simulink model "non_linear_MBS_PF_final"  (R2021b)
settings: Solver=ode15s  StartTime=0  StopTime=SimTime  FixedStep=.00001  RelTol=1e-3
values from neighbouring files (symbols this model uses):
  A_ratio = 1.41    [non_linear_param_MBS_PF_final.m]    (also in non_linear_param_final.m)
  Annulus_area = Bore_area-Rod_area    (= 2356.19449019)    [non_linear_param_MBS_PF_final.m]    (also in non_linear_param_final.m)
  beta_F = 12000e5    (= 1200000000)    [non_linear_param_MBS_PF_final.m]    (also in non_linear_param_final.m)
  Bore_area = pi/4*Bore_diameter^2    (= 3318.30724035)    [non_linear_param_MBS_PF_final.m]    (also in non_linear_param_final.m)
  cyl_c_end = 9e8    (= 900000000)    [non_linear_param_MBS_PF_final.m]    (also in non_linear_param_final.m)
  cyl_c_visc = 1500    [non_linear_param_MBS_PF_final.m]    (also in non_linear_param_final.m)
  cyl_CH_stat = 1/50    (= 0.02)    [non_linear_param_MBS_PF_final.m]    (also in non_linear_param_final.m)
  cyl_F_coul = 75    [non_linear_param_MBS_PF_final.m]    (also in non_linear_param_final.m)
  cyl_FH_stat = 500    [non_linear_param_MBS_PF_final.m]    (also in non_linear_param_final.m)
  cyl_gamma = 250    [non_linear_param_MBS_PF_final.m]    (also in non_linear_param_final.m)
  cyl_k_end = 6e9    (= 6000000000)    [non_linear_param_MBS_PF_final.m]    (also in non_linear_param_final.m)
  cyl_KLi = 0    [non_linear_param_MBS_PF_final.m]    (also in non_linear_param_final.m)
  d_eff_long = sqrt(2*J_sec_long*k_eff_long)    [non_linear_param_MBS_PF_final.m]    (also in non_linear_param_final.m)
  d_eff_short = sqrt(2*J_sec_short*k_eff_short)    [non_linear_param_MBS_PF_final.m]    (also in non_linear_param_final.m)
  DB = 0    [non_linear_param_MBS_PF_final.m]    (also in non_linear_param_final.m = -0.114)
  DR = 1    [non_linear_param_MBS_PF_final.m]    (also in non_linear_param_final.m)
  dr_dcv = 0.7    [non_linear_param_MBS_PF_final.m]    (also in non_linear_param_final.m)
  Eff_Bulk_On = 1    [non_linear_param_MBS_PF_final.m]    (also in non_linear_param_final.m)
  eta_air = 0.7e-2    (= 0.007)    [non_linear_param_MBS_PF_final.m]    (also in non_linear_param_final.m)
  k_cv = 15    [non_linear_param_MBS_PF_final.m]    (also in non_linear_param_final.m)
  k_DCV_PA = 7.85    [non_linear_param_MBS_PF_final.m]    (also in non_linear_param_final.m)
  k_DCV_PB = 7.55    [non_linear_param_MBS_PF_final.m]    (also in non_linear_param_final.m)
  k_DCV_T = 7.85    [non_linear_param_MBS_PF_final.m]    (also in non_linear_param_final.m)
  k_eff_long = E*I/I_sec_long    (= 3050381.94041)    [non_linear_param_MBS_PF_final.m]    (also in non_linear_param_final.m)
  k_eff_short = E*I/I_sec_short    (= 13451432.5427)    [non_linear_param_MBS_PF_final.m]    (also in non_linear_param_final.m)
  k_ocv = 15    [non_linear_param_MBS_PF_final.m]    (also in non_linear_param_final.m)
  K_oil = 1.5e4*0+12000    (= 12000)    [non_linear_param_MBS_PF_final.m]    (also in non_linear_param_final.m)
  k_tanh = 0.005    [non_linear_param_MBS_PF_final.m]    (also in non_linear_param_final.m)
  kappa_air = 1.4    [non_linear_param_MBS_PF_final.m]    (also in non_linear_param_final.m)
  kHP = C*kF_pf_Up    (= 2.90878e-12)    [non_linear_param_MBS_PF_final.m]
  kI = (0.54*ku)/Ti    (= 0.048)    [non_linear_param_MBS_PF_final.m]
  kP = 0.45*ku    (= 0.018)    [non_linear_param_MBS_PF_final.m]
  kq_xS_PB = 4.8946e-04    (= 0.00048946)    [non_linear_param_MBS_PF_final.m]
  KR = 1    [non_linear_param_MBS_PF_final.m]    (also in non_linear_param_final.m)
  mu_ocv = 10    [non_linear_param_MBS_PF_final.m]    (also in non_linear_param_final.m = 3)
  muC = (Bore_diameter^2)/(Bore_diameter^2-Rod_diameter^2)    (= 1.40833333333)    [non_linear_param_MBS_PF_final.m]
  OL_pa = -0.114    [non_linear_param_MBS_PF_final.m]    (also in non_linear_param_final.m)
  OL_pb = 0.114    [non_linear_param_MBS_PF_final.m]    (also in non_linear_param_final.m)
  p0_A = 62.79    [non_linear_param_MBS_PF_final.m]    (also in non_linear_param_final.m)
  p0_A1 = 1.08    [non_linear_param_MBS_PF_final.m]    (also in non_linear_param_final.m)
  p0_B = 1.213    [non_linear_param_MBS_PF_final.m]    (also in non_linear_param_final.m)
  p_cv_fo = 1    [non_linear_param_MBS_PF_final.m]    (also in non_linear_param_final.m)
  p_cv_set = 2.8    [non_linear_param_MBS_PF_final.m]    (also in non_linear_param_final.m)
  p_ocv_fo = 350    [non_linear_param_MBS_PF_final.m]    (also in non_linear_param_final.m)
  p_ocv_set = 196    [non_linear_param_MBS_PF_final.m]    (also in non_linear_param_final.m = 150)
  p_pc_set = 7    [non_linear_param_MBS_PF_final.m]    (also in non_linear_param_final.m)
  p_R = 1.1    [non_linear_param_MBS_PF_final.m]    (also in non_linear_param_final.m)
  p_S = 180    [non_linear_param_MBS_PF_final.m]    (also in non_linear_param_final.m)
  patm = 1.013e5    (= 101300)    [non_linear_param_MBS_PF_final.m]    (also in non_linear_param_final.m)
  Stroke = 500    [non_linear_param_MBS_PF_final.m]    (also in non_linear_param_final.m)
  t0 = 3    [non_linear_param_MBS_PF_final.m]    (also in non_linear_param_final.m = 1)
  tau_ocv = 0.0889*0+0.001    (= 0.001)    [non_linear_param_MBS_PF_final.m]    (also in non_linear_param_final.m)
  tau_pc = tau_ocv    (= 0.001)    [non_linear_param_MBS_PF_final.m]    (also in non_linear_param_final.m)
  tHP = tF_pf_Up/(wn_pf)    (= 0.114613180516)    [non_linear_param_MBS_PF_final.m]
  tStepDown = 4.7*0+7.5    (= 7.5)    [non_linear_param_MBS_PF_final.m]    (also in non_linear_param_final.m = 4.6)
  tStepUp = 10.0    (= 10)    [non_linear_param_MBS_PF_final.m]    (also in non_linear_param_final.m = 6.2)
  tWait = 5.0    (= 5)    [non_linear_param_MBS_PF_final.m]    (also in non_linear_param_final.m = 8)
  UL_at = -0.05    [non_linear_param_MBS_PF_final.m]    (also in non_linear_param_final.m)
  UL_bt = 0.05    [non_linear_param_MBS_PF_final.m]    (also in non_linear_param_final.m)
  Up = 1    [non_linear_param_MBS_PF_final.m]    (also in non_linear_param_final.m)
  uS_sp = -0.4    [non_linear_param_MBS_PF_final.m]    (also in non_linear_param_final.m = -0.55)
  V_CH_A = 1.0e-03    (= 0.001)    [non_linear_param_MBS_PF_final.m]    (also in non_linear_param_final.m)
  V_CH_A1 = 0.1e-03    (= 0.0001)    [non_linear_param_MBS_PF_final.m]    (also in non_linear_param_final.m)
  V_CH_B = 1.25e-03    (= 0.00125)    [non_linear_param_MBS_PF_final.m]    (also in non_linear_param_final.m)
  wn_dcv = 30    [non_linear_param_MBS_PF_final.m]    (also in non_linear_param_final.m)
undefined symbols (not defined in the file or in its folder): sigbuilder_block, smiData, uS0, X, Y, Z
libraries: sm_lib x189, nesl_utility x4, eeGeneralControl x2
(part 1 of 6)
settings Model: Creator=Zygis  LastModifiedBy=danielh
settings SolverCC: ZeroCrossAlgorithm=Adaptive  AlgebraicLoopSolver=TrustRegion
settings DataIOCC: ExternalInput=getinputstruct(report1)  InitialState=getstatestruct(report1)  ReturnWorkspaceOutputs=off  Refine=2
settings DebuggingCC: IgnoredZcDiagnostic=none  UniqueDataStoreMsg=error  SFMachineParentedDataDiag=warning  UnderSpecifiedDimensionMsg=warning  ArithmeticOperatorsInVariantConditions=warning
settings HardwareCC: TargetBitPerPointer=64  TargetBitPerSizeT=64  TargetBitPerPtrDiffT=64  TargetLongLongMode=on  TargetIntDivRoundTo=Zero  TargetEndianess=LittleEndian  TargetWordSize=64  TargetHWDeviceType=MATLAB Host  ProdEqTarget=off
settings SFSimCC: SimUseLocalCustomCode=off  CustomCodeUndefinedFunction=UseInterfaceOnly
settings RTWCC: Description=Rapid Simulation Target  SystemTargetFile=rsim.tlc  TemplateMakefile=rsim_default_tmf  GenerateReport=on  RTWUseLocalCustomCode=off
settings RSimTargetCC: TargetLangStandard=C99 (ISO)
settings DiagnosticsConfigSet: SimMechanicsJointTargetOverSpecification=warning

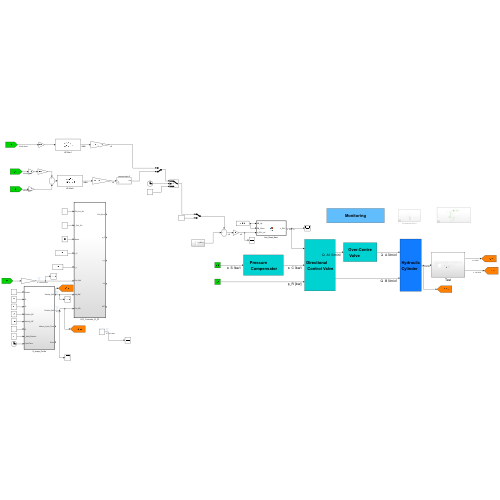
[thumbnail: Simulink's stored preview of the top-level diagram]
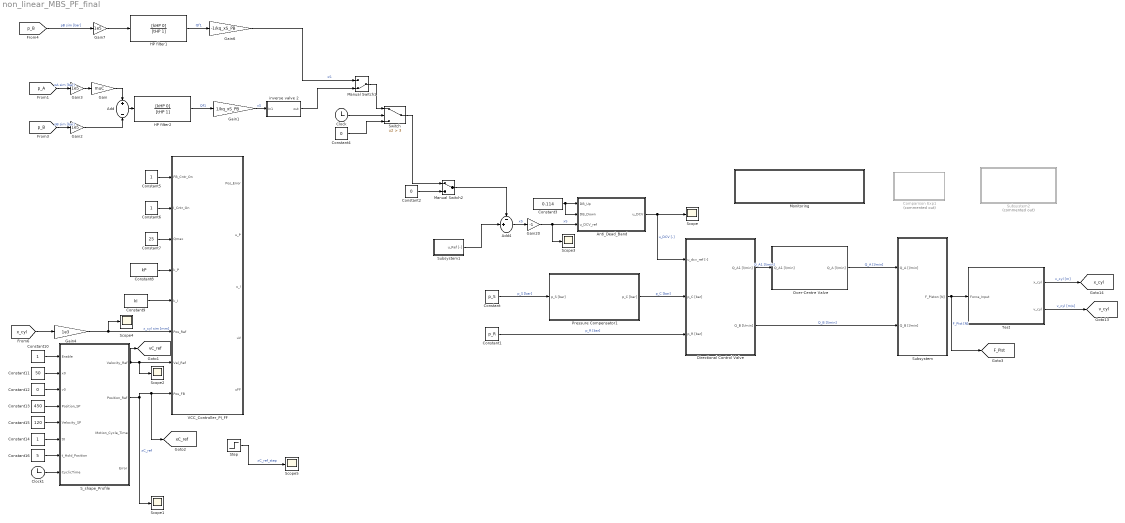
== system non_linear_MBS_PF_final ==
  Add : Sum  Inputs=+-
  Add4 : Sum  Inputs=-+|
  Anti_Dead_Band : MATLAB Function  TreatAsAtomicUnit=on
  Clock : Clock
  Clock1 : Clock
  Comparison Exp1 : Subsystem  Commented=on
  Constant : Constant  Value=p_S
  Constant1 : Constant  Value=p_R
  Constant10 : Constant  OutDataTypeStr=boolean
  Constant11 : Constant  Value=50
  Constant12 : Constant  Value=0
  Constant13 : Constant  Value=450
  Constant14 : Constant
  Constant15 : Constant  Value=120
  Constant16 : Constant  Value=5
  Constant2 : Constant  Value=0
  Constant3 : Constant  Value=0.114
  Constant4 : Constant  Value=0
  Constant5 : Constant  OutDataTypeStr=boolean
  Constant6 : Constant  OutDataTypeStr=boolean
  Constant7 : Constant  Value=25
  Constant8 : Constant  Value=kP  VectorParams1D=off
  Constant9 : Constant  Value=kI
  Directional Control Valve : Subsystem
      mask Display: disp('{\bf\fontsize{22}Directional\newline Control Valve}', 'texmode','on');
  Gain : Gain  Gain=muC
  Gain1 : Gain  Gain=1/kq_xS_PB
  Gain2 : Gain  Gain=1e5
  Gain20 : Gain  Gain=-1
  Gain3 : Gain  Gain=1e5
  Gain4 : Gain  Gain=1e3
  Gain6 : Gain  Gain=-1/kq_xS_PB
  Gain7 : Gain  Gain=1e5
  HP filter1 : TransferFcn  Numerator=[kHP 0]  Denominator=[tHP 1]
  HP filter2 : TransferFcn  Numerator=[kHP 0]  Denominator=[tHP 1]
  Manual Switch1 : ManualSwitch  CurrentSetting=0
  Manual Switch2 : ManualSwitch
  Monitoring : Subsystem
      mask Display: disp('{\bf\fontsize{22}Monitoring}', 'texmode','on');
  Over-Centre Valve : Subsystem
      mask Display: disp('{\bf\fontsize{22}Over-Centre\newline Valve}', 'texmode','on');
  Pressure Compensator1 : Subsystem
      mask Display: disp('{\bf\fontsize{22}Pressure\newline Compensator}', 'texmode','on');
  S_shape_Profile : Subsystem  TreatAsAtomicUnit=on  VariantControl=S_shape_Profile
  Scope{,1..5} (6 alike) : Scope
  Step : Step  Before=50  After=450  SampleTime=0
  Subsystem : Subsystem
      mask Display: disp('{\bf\fontsize{22} Hydraulic \newline Cylinder}', 'texmode','on');
  Subsystem1 : Subsystem
  Subsystem2 : Subsystem  Commented=on
  Switch : Switch  Criteria=u2 > Threshold  Threshold=3
  Test : Subsystem  VariantControl=Flexible_model
  VCC_Controller_PI_FF : Subsystem  TreatAsAtomicUnit=on
  inverse valve 2 : Subsystem  mask{k=5}
  tag scope: F_Pist=global, vC_ref=global, v_cyl=global, xC_ref=global, x_cyl=global
  wires:
  Over-Centre Valve/1(Q_A [l/min]) -> Subsystem/1(Q_A [l/min])  "Q_A [l/min]"
  Directional Control Valve/1(Q_A1 [l/min]) -> Over-Centre Valve/1(Q_A1 [l/min])  "Q_A1 [l/min]"
  Constant/1 -> Pressure Compensator1/1(p_S [bar])  "p_S [bar]"
  Directional Control Valve/2(Q_B [l/min]) -> Subsystem/2(Q_B [l/min])  "Q_B [l/min]"
  Constant1/1 -> Directional Control Valve/3(p_R [bar])  "p_R [bar]"
  Pressure Compensator1/1(p_C [bar]) -> Directional Control Valve/2(p_C [bar])  "p_C [bar]"
  Test/1(x_cyl) -> [x_cyl]  "x_cyl [m]"
  Test/2(v_cyl) -> [v_cyl]  "v_cyl [m/s]"
  Subsystem/1(F_Piston [N]) -> [F_Pist], Test/1(Force_Input)  "F_Pist [N]"
  Manual Switch2/1 -> Add4/1
  Constant2/1 -> Manual Switch2/2
  Gain1/1 -> inverse valve 2/1(In1)  "xS"
  HP filter2/1 -> Gain1/1  "Qf1"
  [p_A] -> Gain3/1  "pA sim [bar]"
  Add/1 -> HP filter2/1
  Gain/1 -> Add/1
  [p_B] -> Gain2/1  "pB sim [bar]"
  Add4/1 -> Gain20/1  "xS"
  Anti_Dead_Band/1(u_DCV) -> Scope/1, Directional Control Valve/1(u_dcv_ref [-])  "u_DCV [-]"
  Constant3/1 -> Anti_Dead_Band/2(DB_Down), Anti_Dead_Band/1(DB_Up)
  Gain2/1 -> Add/2
  Gain3/1 -> Gain/1
  Switch/1 -> Manual Switch2/1
  Clock/1 -> Switch/2
  Constant4/1 -> Switch/3
  S_shape_Profile/2(Position_Ref) -> Scope1/1, [xC_ref], VCC_Controller_PI_FF/8(Pos_FB)  "xC_ref"
  [x_cyl] -> Gain4/1
  Gain4/1 -> Scope4/1, VCC_Controller_PI_FF/6(Pos_Ref)  "x_cyl sim [mm]"
  Constant5/1 -> VCC_Controller_PI_FF/1(FB_Cntr_On)
  Constant6/1 -> VCC_Controller_PI_FF/2(I_Cntr_On)
  Constant7/1 -> VCC_Controller_PI_FF/3(Qmax)
  Constant8/1 -> VCC_Controller_PI_FF/4(k_P)
  Constant9/1 -> VCC_Controller_PI_FF/5(k_I)
  Constant10/1 -> S_shape_Profile/1(Enable)
  Constant13/1 -> S_shape_Profile/4(Position_SP)
  Constant12/1 -> S_shape_Profile/3(v0)
  Constant11/1 -> S_shape_Profile/2(x0)
  Constant14/1 -> S_shape_Profile/6(t0)
  Constant15/1 -> S_shape_Profile/5(Velocity_SP)
  Constant16/1 -> S_shape_Profile/7(t_Hold_Position)
  Clock1/1 -> S_shape_Profile/8(CyclicTime)
  Gain20/1 -> Scope3/1, Anti_Dead_Band/3(u_DCV_ref)  "xS"
  Step/1 -> Scope5/1  "xC_ref_step"
  S_shape_Profile/1(Velocity_Ref) -> Scope2/1, VCC_Controller_PI_FF/7(Vel_Ref), [vC_ref]
  [p_B] -> Gain7/1  "pB sim [bar]"
  HP filter1/1 -> Gain6/1  "Qf1"
  Gain7/1 -> HP filter1/1
  inverse valve 2/1(out) -> Manual Switch1/2
  Gain6/1 -> Manual Switch1/1  "xS"
  Manual Switch1/1 -> Switch/1
  Subsystem1/1(u_Ref [-]) -> Add4/2

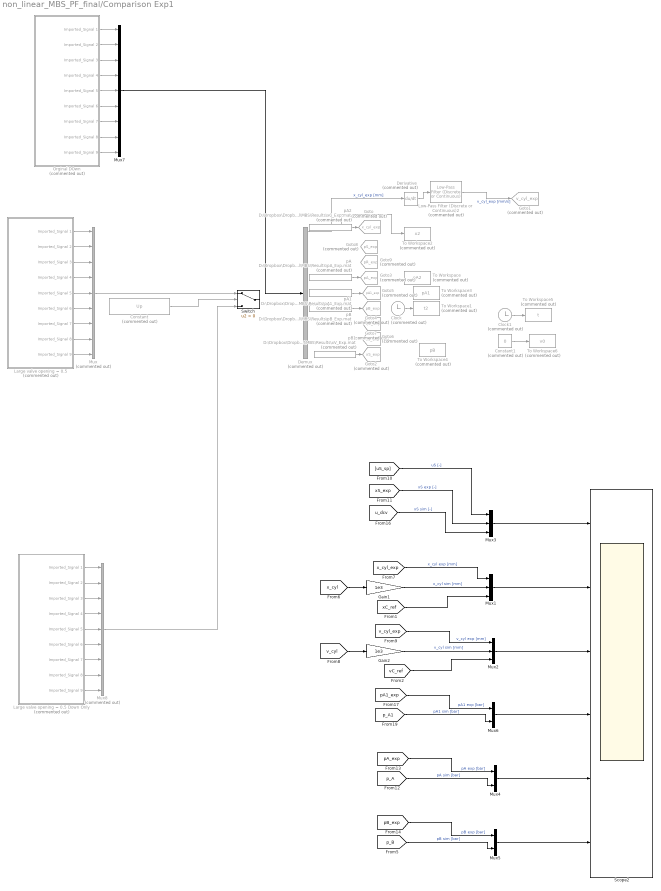
== system non_linear_MBS_PF_final/Comparison Exp1 ==
  Clock : Clock  Commented=on
  Clock1 : Clock  Commented=on
  Constant : Constant  Commented=on  Value=Up
  Constant1 : Constant  Commented=on  Value=0
  Demux : Demux  Commented=on  Outputs=9
  Derivative : Derivative  Commented=on
  Gain1 : Gain  Gain=1e3
  Gain2 : Gain  Gain=1e3
  Goto : Goto  Commented=on  GotoTag=x_cyl_exp  TagVisibility=global
  Goto1 : Goto  Commented=on  GotoTag=v_cyl_exp  TagVisibility=global
  Goto2 : Goto  Commented=on  GotoTag=xS_exp  TagVisibility=global
  Goto3 : Goto  Commented=on  GotoTag=pA_exp  TagVisibility=global
  Goto4 : Goto  Commented=on  GotoTag=pB_exp  TagVisibility=global
  Goto5 : Goto  Commented=on  GotoTag=pA1_exp  TagVisibility=global
  Goto6 : Goto  Commented=on  GotoTag=uS_exp  TagVisibility=global
  Goto7 : Goto  Commented=on  GotoTag=QB_exp  TagVisibility=global
  Goto8 : Goto  Commented=on  GotoTag=pS_exp  TagVisibility=global
  Goto9 : Goto  Commented=on  GotoTag=pR_exp  TagVisibility=global
  Large valve opening = 0.5 : Subsystem  Tag=STV Subsys  Commented=on  LoadFcn=sigbuilder_block('load');  StartFcn=sigbuilder_block('start');  StopFcn=sigbuilder_block('stop');  ClipboardFcn=sigbuilder_block('clipboard');  mask(Sigbuilder block){tuvar=[0:10]}
      mask help: Simulink:masks:SigbuilderBlock_MD
      mask init:
      | if ~strcmp(get_param(bdroot(gcbh),'SimulationStatus'),'stopped') tuvar = sigbuilder_block('maskInit'); end
      settings: CopyFcn=sigbuilder_block('copy');  DeleteFcn=sigbuilder_block('delete');  ModelCloseFcn=sigbuilder_block('modelClose');  PreSaveFcn=sigbuilder_block('preSave');  PauseFcn=sigbuilder_block('pause');  ContinueFcn=sigbuilder_block('continue');  NameChangeFcn=sigbuilder_block('namechange');  OpenFcn=sigbuilder_block('open',[41.25 0 1392.75 801.75 ]);  CloseFcn=sigbuilder_block('close');
      mask Display: plot(0, 0, 100, 100,[2, 2, 32, 32, 2], [68, 8, 8, 68, 68],[32, 2], [38, 38], [32, 19, 2],[53, 60, 44], [32, 17, 17, 2],[16, 16, 31, 31]);txt = getActiveGroup(gcbh);text(2, 100, txt,'verticalAlignment', 'top');
  Large valve opening = 0.5 Down Only : Subsystem  Tag=STV Subsys  Commented=on  LoadFcn=sigbuilder_block('load');  StartFcn=sigbuilder_block('start');  StopFcn=sigbuilder_block('stop');  ClipboardFcn=sigbuilder_block('clipboard');  mask(Sigbuilder block){tuvar=[0:10]}
      mask help: Simulink:masks:SigbuilderBlock_MD
      mask init:
      | if ~strcmp(get_param(bdroot(gcbh),'SimulationStatus'),'stopped') tuvar = sigbuilder_block('maskInit'); end
      settings: CopyFcn=sigbuilder_block('copy');  DeleteFcn=sigbuilder_block('delete');  ModelCloseFcn=sigbuilder_block('modelClose');  PreSaveFcn=sigbuilder_block('preSave');  PauseFcn=sigbuilder_block('pause');  ContinueFcn=sigbuilder_block('continue');  NameChangeFcn=sigbuilder_block('namechange');  OpenFcn=sigbuilder_block('open',[41.25 0 1392.75 801.75 ]);  CloseFcn=sigbuilder_block('close');
      mask Display: plot(0, 0, 100, 100,[2, 2, 32, 32, 2], [68, 8, 8, 68, 68],[32, 2], [38, 38], [32, 19, 2],[53, 60, 44], [32, 17, 17, 2],[16, 16, 31, 31]);txt = getActiveGroup(gcbh);text(2, 100, txt,'verticalAlignment', 'top');
  Low-Pass Filter (Discrete or Continuous)2 : eeGeneralControl/Low-Pass Filter (Discrete or Continuous)  Commented=on  K=1  T=0.05  B=150  A=-150  init_option=Inherited from block input  x0=0  Ts=0.001
  Mux : Mux  Commented=on  Inputs=9
  Mux1 : Mux  Inputs=3
  Mux2 : Mux  Inputs=3
  Mux3 : Mux  Inputs=3
  Mux4 : Mux  Inputs=2
  Mux5 : Mux  Inputs=2
  Mux6 : Mux  Inputs=2
  Mux7 : Mux  Inputs=9
  Mux8 : Mux  Commented=on  Inputs=9
  Orginal DOwn : Subsystem  Tag=STV Subsys  Commented=on  LoadFcn=sigbuilder_block('load');  StartFcn=sigbuilder_block('start');  StopFcn=sigbuilder_block('stop');  ClipboardFcn=sigbuilder_block('clipboard');  mask(Sigbuilder block){tuvar=[0:10]}
      mask help: Simulink:masks:SigbuilderBlock_MD
      mask init:
      | if ~strcmp(get_param(bdroot(gcbh),'SimulationStatus'),'stopped') tuvar = sigbuilder_block('maskInit'); end
      settings: CopyFcn=sigbuilder_block('copy');  DeleteFcn=sigbuilder_block('delete');  ModelCloseFcn=sigbuilder_block('modelClose');  PreSaveFcn=sigbuilder_block('preSave');  PauseFcn=sigbuilder_block('pause');  ContinueFcn=sigbuilder_block('continue');  NameChangeFcn=sigbuilder_block('namechange');  OpenFcn=sigbuilder_block('open',[437.6 199.2 550.4 400 ]);  CloseFcn=sigbuilder_block('close');
      mask Display: plot(0, 0, 100, 100,[2, 2, 32, 32, 2], [68, 8, 8, 68, 68],[32, 2], [38, 38], [32, 19, 2],[53, 60, 44], [32, 17, 17, 2],[16, 16, 31, 31]);txt = getActiveGroup(gcbh);text(2, 100, txt,'verticalAlignment', 'top');
  Scope2 : Scope  NumInputPorts=6
  Switch : Switch  Criteria=u2 > Threshold
  To Workspace : ToWorkspace  Commented=on  VariableName=pA2  SampleTime=0.001
      settings: Save2DSignal=2-D array (concatenate along first dimension)
  To Workspace1 : ToWorkspace  Commented=on  VariableName=t2  SampleTime=0.001
      settings: Save2DSignal=2-D array (concatenate along first dimension)
  To Workspace2 : ToWorkspace  Commented=on  VariableName=x2  SampleTime=0.001
      settings: Save2DSignal=2-D array (concatenate along first dimension)
  To Workspace3 : ToWorkspace  Commented=on  VariableName=pA1  SampleTime=0.001
      settings: Save2DSignal=2-D array (concatenate along first dimension)
  To Workspace4 : ToWorkspace  Commented=on  VariableName=pB  SampleTime=0.001
      settings: Save2DSignal=2-D array (concatenate along first dimension)
  To Workspace5 : ToWorkspace  Commented=on  VariableName=t  SampleTime=0.001
      settings: Save2DSignal=2-D array (concatenate along first dimension)
  To Workspace6 : ToWorkspace  Commented=on  VariableName=v0  SampleTime=0.001
      settings: Save2DSignal=2-D array (concatenate along first dimension)
  pA : FromFile  Commented=on  FileName=D:\Dropbox\Dropbox\PhD\Models\LSS model\Comparison_Paper_Model\Non Linear Model\MBS\Results\pA_Exp.mat  SampleTime=0
  pA1 : FromFile  Commented=on  FileName=D:\Dropbox\Dropbox\PhD\Models\LSS model\Comparison_Paper_Model\Non Linear Model\MBS\Results\pA1_Exp.mat  SampleTime=0
  pA2 : FromFile  Commented=on  FileName=D:\Dropbox\Dropbox\PhD\Models\LSS model\Comparison_Paper_Model\Non Linear Model\MBS\Results\xC_Exp.mat  SampleTime=0
  pB : FromFile  Commented=on  FileName=D:\Dropbox\Dropbox\PhD\Models\LSS model\Comparison_Paper_Model\Non Linear Model\MBS\Results\pB_Exp.mat  SampleTime=0
  pB1 : FromFile  Commented=on  FileName=D:\Dropbox\Dropbox\PhD\Models\LSS model\Comparison_Paper_Model\Non Linear Model\MBS\Results\xV_Exp.mat  SampleTime=0
  wires:
  Demux/1 -> To Workspace2/1, Derivative/1  "x_cyl_exp [mm]"
  Low-Pass Filter (Discrete or Continuous)2/1 -> Goto1/1  "v_cyl_exp [mm/s]"
  Derivative/1 -> Low-Pass Filter (Discrete or Continuous)2/1
  Large valve opening = 0.5/4(Imported_Signal 4) -> Mux/4
  Large valve opening = 0.5/5(Imported_Signal 5) -> Mux/5
  Large valve opening = 0.5/6(Imported_Signal 6) -> Mux/6
  Large valve opening = 0.5/7(Imported_Signal 7) -> Mux/7
  Large valve opening = 0.5/8(Imported_Signal 8) -> Mux/8
  Large valve opening = 0.5/9(Imported_Signal 9) -> Mux/9
  Large valve opening = 0.5/3(Imported_Signal 3) -> Mux/3
  Large valve opening = 0.5/1(Imported_Signal 1) -> Mux/1
  Large valve opening = 0.5/2(Imported_Signal 2) -> Mux/2
  Mux/1 -> Switch/1
  Constant/1 -> Switch/2
  [x_cyl] -> Gain1/1
  [p_A] -> Mux4/2  "pA sim [bar]"
  [v_cyl] -> Gain2/1
  Mux4/1 -> Scope2/5
  Mux2/1 -> Scope2/3
  [xS_exp] -> Mux3/2  "xS exp [-]"
  [pA_exp] -> Mux4/1  "pA exp [bar]"
  Gain1/1 -> Mux1/2  "x_cyl sim [mm]"
  Gain2/1 -> Mux2/2  "v_cyl sim [mm]"
  [p_A1] -> Mux6/2  "pA1 sim [bar]"
  [pB_exp] -> Mux5/1  "pB exp [bar]"
  [u_dcv] -> Mux3/3  "xS sim [-]"
  Mux3/1 -> Scope2/1
  [uS_sp] -> Mux3/1  "uS [-]"
  [p_B] -> Mux5/2  "pB sim [bar]"
  Mux6/1 -> Scope2/4
  Mux1/1 -> Scope2/2
  [v_cyl_exp] -> Mux2/1  "v_cyl exp [mm]"
  [pA1_exp] -> Mux6/1  "pA1 exp [bar]"
  Mux5/1 -> Scope2/6
  Large valve opening = 0.5 Down Only/4(Imported_Signal 4) -> Mux8/4
  Large valve opening = 0.5 Down Only/8(Imported_Signal 8) -> Mux8/8
  Large valve opening = 0.5 Down Only/1(Imported_Signal 1) -> Mux8/1
  Large valve opening = 0.5 Down Only/2(Imported_Signal 2) -> Mux8/2
  Large valve opening = 0.5 Down Only/6(Imported_Signal 6) -> Mux8/6
  Large valve opening = 0.5 Down Only/9(Imported_Signal 9) -> Mux8/9
  Large valve opening = 0.5 Down Only/3(Imported_Signal 3) -> Mux8/3
  Large valve opening = 0.5 Down Only/5(Imported_Signal 5) -> Mux8/5
  Large valve opening = 0.5 Down Only/7(Imported_Signal 7) -> Mux8/7
  Mux8/1 -> Switch/3
  Orginal DOwn/1(Imported_Signal 1) -> Mux7/1
  Orginal DOwn/8(Imported_Signal 8) -> Mux7/8
  Orginal DOwn/2(Imported_Signal 2) -> Mux7/2
  Orginal DOwn/9(Imported_Signal 9) -> Mux7/9
  Orginal DOwn/6(Imported_Signal 6) -> Mux7/6
  Orginal DOwn/5(Imported_Signal 5) -> Mux7/5
  Orginal DOwn/3(Imported_Signal 3) -> Mux7/3
  Orginal DOwn/7(Imported_Signal 7) -> Mux7/7
  Orginal DOwn/4(Imported_Signal 4) -> Mux7/4
  Clock/1 -> To Workspace1/1
  Mux7/1 -> Demux/1
  Clock1/1 -> To Workspace5/1
  Constant1/1 -> To Workspace6/1
  [x_cyl_exp] -> Mux1/1  "x_cyl exp [mm]"
  pA/1 -> Goto3/1
  pA1/1 -> Goto5/1
  pB/1 -> Goto4/1
  pB1/1 -> Goto2/1
  pA2/1 -> Goto/1
  [xC_ref] -> Mux1/3
  [vC_ref] -> Mux2/3
